simulink model "fft_16k_core"  (Simulink 7.4)
settings: Solver=ode45  StartTime=0.0  StopTime=10.0  RelTol=1e-3
libraries: xbsIndex_r4 x2252, casper_library_misc x100, casper_library_delays x17, casper_library_ffts_twiddle x1
(part 14 of 41)

== system fft_16k_core/fft_wideband_real/fft_direct/butterfly2_1/twiddle_general_4mult ==
  a : Inport
  b : Inport  Port=2
  sync : Inport  Port=3
  AddSub : xbsIndex_r4/AddSub  mode=Subtraction  use_carryin=off  use_carryout=off  en=off  latency=add_latency  precision=Full  arith_type=Unsigned  n_bits=16  bin_pt=14  quantization=Truncate  overflow=Wrap  dbl_ovrd=off  use_behavioral_HDL=on  hw_selection=Fabric  pipelined=off  block_type=addsub
  AddSub1 : xbsIndex_r4/AddSub  mode=Addition  use_carryin=off  use_carryout=off  en=off  latency=add_latency  precision=Full  arith_type=Unsigned  n_bits=16  bin_pt=14  quantization=Truncate  overflow=Wrap  dbl_ovrd=off  use_behavioral_HDL=on  hw_selection=Fabric  pipelined=off  block_type=addsub
  c_to_ri1 : casper_library_misc/c_to_ri  SystemSampleTime=-1  n_bits=input_bit_width  bin_pt=input_bit_width-1
  c_to_ri2 : casper_library_misc/c_to_ri  SystemSampleTime=-1  n_bits=coeff_bit_width  bin_pt=coeff_bit_width-2
      settings: AttributesFormatString=18_16 r/i
  c_to_ri3 : casper_library_misc/c_to_ri  SystemSampleTime=-1  n_bits=input_bit_width  bin_pt=input_bit_width-1
  coeff_gen : Subsystem  AncestorBlock=casper_library_ffts_twiddle_coeff_gen/coeff_gen  mask(coeff_gen){Coeffs=[6.123233995736766035868820147292e-17-1i     -0.00038349518757137469231535686908785-0.99999992646571789212117664646939i     -0.00076699031874272389271846028790947-0.99999970586288222662574298738036i      -0.0011504853371136860608336949240993-0.99999933819152553304832053981954i      -0.0015339801862846436358162049984344-0.99999882345170187925020854891045i       -0.001917474809855337480185566434443-0.99999816164348698244168645032914i      -0.0023009691514257637860729754919475-0.99999735276697820918201387030422i       -0.0026844631545959608126539208683425-0.9999963968222943533348257005855i       -0.003067956762966015608201653108722-0.99999529380957619117964441102231i      -0.0034514499201358517233018119441113-0.99999404372898581527806527446955i      -0.0038349425697061256292086373065331-0.99999264658070718958526867936598i      -0.0042184346552769013891093230483875-0.99999110236494559433850781715591i      -0.0046019261204485496785654419227285-0.99998941108192840321322591989883i      -0.0049854169088215296440358414997718-0.99998757273190408412233409762848i      -0.0053689069639961803023786579558418-0.99998558731514319841693350099376i      -0.0057523962295736143571223131232273-0.99998345483193773475250054616481i      -0.0061358846491543928697298682095607-0.99998117528260110908888691483298i       -0.0065193721663394264484447937491041-0.9999787486674688308241343293048i      -0.0069028587247297540710477825598446-0.99997617498689761461605485237669i       -0.0072863442679265604320915095115652-0.9999734542412659354937431999133i      -0.0076698287395309547656574444829403-0.99997058643097413987987920336309i      -0.0080533120831448694321164083476106-0.99996757155644377945691303466447i      -0.0084367942423697380588398786471771-0.99996440961811827730087998133968i       -0.008820275160807390657513593623662-0.99996110061646281685909798397915i      -0.0092037547820598367837030551186217-0.99995764455196389786095778617891i      -0.0095872330497290617068451012983132-0.99995404142512978040713278460316i       -0.0099707099074179059150502268948912-0.9999502912364904849695790289843i       -0.010354185298728760603048648647473-0.99994639398659745932462783457595i       -0.010737659167264448911716101520142-0.99994234967602391161989316969994i       -0.011121131456628019495980197461904-0.99993815830536458832966673071496i       -0.011504602110422753463714329313916-0.99993381987523599629952286704793i       -0.011888071072251949270026649685406-0.99992933438627606967941119364696i       -0.012271538285719823038744102916553-0.99992470183914450299056397852837i       -0.012655003694430179764229826844257-0.99991992223452275112549614277668i       -0.013038467241987310163420232811404-0.99991499557311347423649294796633i       -0.013421928871995784243731364426822-0.99990992185564153693633215880254i       -0.013805388528060225789007020580357-0.99990470108285289807525941796484i       -0.014188846153786221354620167289795-0.99989933325551538789710548371659i       -0.014572301692778981060949483605782-0.99989381837441848599468130487367i        -0.01495575508864425452737467736597-0.99988815644037332130977802080452i       -0.015339206284988098419330704302865-0.99988234745421256111086449891445i       -0.015722655225416895530266003788711-0.99987639141679041099308733464568i       -0.016106101853537141410654953688208-0.99987028832898294794517823902424i       -0.016489546112956332546417570483754-0.99986403819168767626024418859743i        -0.01687298794728165143852471885566-0.99985764100582386060267481298069i       -0.017256427300120856516141287784194-0.99985109677233219294123500731075i       -0.017639864115082073969809073332726-0.99984440549217523663827478230814i        -0.018023298335773579176288805570039-0.9998375671663370933828218767303i       -0.018406729905804695285320704556398-0.99983058179582340319058175737155i       -0.018790158768784474829782737970163-0.99982344938166156644854254409438i       -0.019173584868322577495769465372177-0.99981616992490041084806762228254i ...(+319547 chars)], coeff_bit_width=coeff_bit_width, StepPeriod=0, bram_latency=bram_latency, coeffs_bram=on}
      mask prompts: Coeffs "Coefficients: (0 to 2^(FFTSize-1)-1)"; coeff_bit_width "Coefficient Bit Width:"; StepPeriod "Step Period: (2^?)"; bram_latency "Latency:"; coeffs_bram "Store coefficients in BRAM"
      mask help: Generates a sequence of complex coefficients
      mask init:
      | coeff_gen_init( gcb, ...
      |     'Coeffs', Coeffs, ...
      |     'coeff_bit_width', coeff_bit_width, ...
      |     'StepPeriod', StepPeriod, ...
      |     'bram_latency', bram_latency, ...
      |     'coeffs_bram', coeffs_bram);
      settings: AttributesFormatString=4096 @ (18,16)
      mask CallbackString:
      | Coeffs = eval(get_param(gcb, 'Coeffs'));
      | if( length(Coeffs) == 1 ),
      |     set_param(gcb, 'MaskVisibilities',{'on','on','off','off','off'});
      | else
      |     set_param(gcb, 'MaskVisibilities',{'on','on','on','on','on'});
      | end||||
  convert0 : xbsIndex_r4/Convert  arith_type="Signed  (2's comp)"  n_bits=input_bit_width+4  bin_pt=input_bit_width+1  quantization="Round  (unbiased: +/- Inf)"  overflow=Wrap  latency=conv_latency  dbl_ovrd=off  pipeline=on  block_type=convert
      settings: block_version=11.4
  convert1 : xbsIndex_r4/Convert  arith_type="Signed  (2's comp)"  n_bits=input_bit_width+4  bin_pt=input_bit_width+1  quantization="Round  (unbiased: +/- Inf)"  overflow=Wrap  latency=conv_latency  dbl_ovrd=off  pipeline=on  block_type=convert
      settings: block_version=11.4
  delay0 : xbsIndex_r4/Delay  en=off  latency=mult_latency + add_latency + bram_latency + conv_latency  dbl_ovrd=off  reg_retiming=off  block_type=delay
      settings: block_version=11.4
  delay1 : xbsIndex_r4/Delay  en=off  latency=bram_latency  dbl_ovrd=off  reg_retiming=on  block_type=delay
      settings: block_version=11.4
  delay2 : xbsIndex_r4/Delay  en=off  latency=mult_latency + add_latency + bram_latency + conv_latency  dbl_ovrd=off  reg_retiming=off  block_type=delay
      settings: block_version=11.4
  mult{,1..3} (4 alike) : xbsIndex_r4/Mult  precision=Full  arith_type="Signed  (2's comp)"  n_bits=16  bin_pt=14  quantization=Truncate  overflow=Wrap  en=off  latency=mult_latency  dbl_ovrd=off  use_behavioral_HDL=on  opt=Speed  use_embedded=off  optimum_pipeline=off  pipeline=on  use_rpm=on  placement_style=Triangular  block_type=mult
      settings: block_version=11.4
  a_re : Outport
  a_im : Outport  Port=2
  bw_re : Outport  Port=3
  bw_im : Outport  Port=4
  sync_out : Outport  Port=5
  wires:
  a/1 -> delay0/1
  delay0/1 -> c_to_ri1/1
  c_to_ri1/1 -> a_re/1
  c_to_ri1/2 -> a_im/1
  sync/1 -> delay2/1, coeff_gen/1(rst)
  delay2/1 -> sync_out/1
  coeff_gen/1(w) -> c_to_ri2/1
  b/1 -> delay1/1
  delay1/1 -> c_to_ri3/1
  c_to_ri3/1 -> mult/1, mult3/1
  c_to_ri2/1 -> mult/2, mult1/2
  c_to_ri3/2 -> mult1/1, mult2/1
  c_to_ri2/2 -> mult2/2, mult3/2
  mult/1 -> AddSub/1
  mult1/1 -> AddSub1/1
  mult2/1 -> AddSub/2
  mult3/1 -> AddSub1/2
  AddSub/1 -> convert0/1
  convert0/1 -> bw_re/1
  AddSub1/1 -> convert1/1
  convert1/1 -> bw_im/1
